simulink model "spec2in_8192ch_1080mhz"  (R2013b) (continued)
== system spec2in_8192ch_1080mhz/fft_wideband_real0/fft_direct == (continued)
  butterfly3_2 : Subsystem  AncestorBlock=casper_library_ffts/butterfly_direct  mask(butterfly_direct){n_inputs=1, biplex=off, FFTSize=14, Coeffs=[ 2 4098 2050 6146 1026 5122 3074 7170 514 4610 2562 6658 1538 5634 3586 7682 258 4354 2306 6402 1282 5378 3330 7426 770 4866 2818 6914 1794 5890 3842 7938 130 4226 2178 6274 1154 5250 3202 7298 642 4738 2690 6786 1666 5762 3714 7810 386 4482 2434 6530 1410 5506 3458 7554 898 4994 2946 7042 1922 6018 3970 8066 66 4162 2114 6210 1090 5186 3138 7234 578 4674 2626 6722 1602 5698 3650 7746 322 4418 2370 6466 1346 5442 3394 7490 834 4930 2882 6978 1858 5954 3906 8002 194 4290 2242 6338 1218 5314 3266 7362 706 4802 2754 6850 1730 5826 3778 7874 450 4546 2498 6594 1474 5570 3522 7618 962 5058 3010 7106 1986 6082 4034 8130 34 4130 2082 6178 1058 5154 3106 7202 546 4642 2594 6690 1570 5666 3618 7714 290 4386 2338 6434 1314 5410 3362 7458 802 4898 2850 6946 1826 5922 3874 7970 162 4258 2210 6306 1186 5282 3234 7330 674 4770 2722 6818 1698 5794 3746 7842 418 4514 2466 6562 1442 5538 3490 7586 930 5026 2978 7074 1954 6050 4002 8098 98 4194 2146 6242 1122 5218 3170 7266 610 4706 2658 6754 1634 5730 3682 7778 354 4450 2402 6498 1378 5474 3426 7522 866 4962 2914 7010 1890 5986 3938 8034 226 4322 2274 6370 1250 5346 3298 7394 738 4834 2786 6882 1762 5858 3810 7906 482 4578 2530 6626 1506 5602 3554 7650 994 5090 3042 7138 2018 6114 4066 8162 18 4114 2066 6162 1042 5138 3090 7186 530 4626 2578 6674 1554 5650 3602 7698 274 4370 2322 6418 1298 5394 3346 7442 786 4882 2834 6930 1810 5906 3858 7954 146 4242 2194 6290 1170 5266 3218 7314 658 4754 2706 6802 1682 5778 3730 7826 402 4498 2450 6546 1426 5522 3474 7570 914 5010 2962 7058 1938 6034 3986 8082 82 4178 2130 6226 1106 5202 3154 7250 594 4690 2642 6738 1618 5714 3666 7762 338 4434 2386 6482 1362 5458 3410 7506 850 4946 2898 6994 1874 5970 3922 8018 210 4306 2258 6354 1234 5330 3282 7378 722 4818 2770 6866 1746 5842 3794 7890 466 4562 2514 6610 1490 5586 3538 7634 978 5074 3026 7122 2002 6098 4050 8146 50 4146 2098 6194 1074 5170 3122 7218 562 4658 2610 6706 1586 5682 3634 7730 306 4402 2354 6450 1330 5426 3378 7474 818 4914 2866 6962 1842 5938 3890 7986 178 4274 2226 6322 1202 5298 3250 7346 690 4786 2738 6834 1714 5810 3762 7858 434 4530 2482 6578 1458 5554 3506 7602 946 5042 2994 7090 1970 6066 4018 8114 114 4210 2162 6258 1138 5234 3186 7282 626 4722 2674 6770 1650 5746 3698 7794 370 4466 2418 6514 1394 5490 3442 7538 882 4978 2930 7026 1906 6002 3954 8050 242 4338 2290 6386 1266 5362 3314 7410 754 4850 2802 6898 1778 5874 3826 7922 498 4594 2546 6642 1522 5618 3570 7666 1010 5106 3058 7154 2034 6130 4082 8178 10 4106 2058 6154 1034 5130 3082 7178 522 4618 2570 6666 1546 5642 3594 7690 266 4362 2314 6410 1290 5386 3338 7434 778 4874 2826 6922 1802 5898 3850 7946 138 4234 2186 6282 1162 5258 3210 7306 650 4746 2698 6794 1674 5770 3722 7818 394 4490 2442 6538 1418 5514 3466 7562 906 5002 2954 7050 1930 6026 3978 8074 74 4170 2122 6218 1098 5194 3146 7242 586 4682 2634 6730 1610 5706 3658 7754 330 4426 2378 6474 1354 5450 3402 7498 842 4938 2890 6986 1866 5962 3914 8010 202 4298 2250 6346 1226 5322 3274 7370 714 4810 2762 6858 1738 5834 3786 7882 458 4554 2506 6602 1482 5578 3530 7626 970 5066 3018 7114 1994 6090 4042 8138 42 4138 2090 6186 1066 5162 3114 7210 554 4650 2602 6698 1578 5674 3626 7722 298 4394 2346 6442 1322 5418 3370 7466 810 4906 2858 6954 1834 5930 3882 7978 170 4266 2218 6314 1194 5290 3242 7338 682 4778 2730 6826 1706 5802 3754 7850 426 4522 2474 6570 1450 5546 3498 7594 938 5034 2986 7082 1962 6058 4010 8106 106 4202 2154 6250 1130 5226 3178 7274 618 4714 2666 6762 1642 5738 3690 7786 362 4458 2410 6506 1386 5482 3434 7530 874 4970 2922 7018 1898 5994 3946 8042 234 4330 2282 6378 1258 5354 3306 7402 746 4842 2794 6890 1770 5866 3818 7914 490 4586 2538 6634 1514 5610 3562 7658 1002 5098 3050 7146 2026 6122 4074 8170 26 4122 2074 6170 1050 5146 3098 7194 538 4634 2586 6682 1562 5658 3610 7706 282 4378 2330 6426 1306 5402 3354 7450 794 4890 2842 6938 1818 5914 3866 7962 154 4250 2202 6298 1178 5274 3226 7322 666 4762 2714 6810 1690 5786 3738 7834 410 4506 2458 6554 1434 5530 ...(+984 chars)], StepPeriod=0, coeff_bit_width=18, input_bit_width=18, bin_pt_in=17, downshift=off, async=off, add_latency=1, mult_latency=2, bram_latency=2, conv_latency=1, quantization=Round  (unbiased: +/- Inf), overflow=Wrap, coeffs_bit_limit=9, coeff_sharing=off, coeff_decimation=off, coeff_generation=off, cal_bits=1, n_bits_rotation=25, max_fanout=4, use_hdl=on, use_embedded=off, bitgrowth=off, hardcode_shifts=off, dsp48_adders=off}
      mask prompts: n_inputs "number inputs"; biplex "Used in biplex FFT"; FFTSize "Size of FFT: (2^? pts)"; Coeffs "Coefficients: (0 to 2^(FFTSize-1)-1)"; StepPeriod "Coefficient Step Period: (2^?)"; coeff_bit_width "Coefficient Bit Width"; input_bit_width "Input Bit Width"; bin_pt_in "Input binary point"; async "asynchronous operation"; add_latency "Add Latency"; mult_latency "Mult Latency"; bram_latency "BRAM Latency"; conv_latency "Convert latency"; quantization "Quantization Behavior"; overflow "Overflow Behavior"; coeffs_bit_limit "Number bits above which to store stage's coefficients in BRAM (2^? bits)"; coeff_sharing "BRAM sharing in coeff storage"; coeff_decimation "Store a fraction of coeff factors where useful"; coeff_generation "Generate coeffs with multipliers where useful"; cal_bits "Number calibration locations when generating coeffs (2^?)"; n_bits_rotation "Feedback rotation vector resolution"; max_fanout "Maximum fanout"; use_hdl "Use behavioural HDL for multipliers"; use_embedded "Use embedded multipliers"; bitgrowth "Bit growth instead of shifting"; hardcode_shifts "Hardcode shift schedule"; dsp48_adders "Use DSP48s for adders"
      mask help: FFT internal block.
      mask init: same as spec2in_8192ch_1080mhz/fft_wideband_real0/fft_direct/butterfly0_0
      settings: AttributesFormatString=twiddle_general
      mask TypeOptions quantization: Truncate | Round  (unbiased: +/- Inf) | Round  (unbiased: Even Values)
      mask TypeOptions overflow: Wrap | Saturate | Error
      mask Callback use_hdl:
      | % make mutually exclusive with use_embedded.
      | 
      | use_hdl = get_param(gcb, 'use_hdl');
      | if strcmp(use_hdl, 'on'),
      |     set_param_state(gcb, 'use_embedded', 'off');
      | else
      |     set_param_state(gcb, 'use_embedded', 'on');
      | end
      mask Callback use_embedded:
      | % make mutually exclusive with use_hdl.
      | 
      | use_hdl = get_param(gcb, 'use_embedded');
      | if strcmp(use_hdl, 'on'),
      |     set_param_state(gcb, 'use_hdl', 'off');
      | else
      |     set_param_state(gcb, 'use_hdl', 'on');
      | end
      mask Callback hardcode_shifts:
      | hardcode_shifts = get_param(gcb, 'hardcode_shifts');
      | set_param_state(gcb, 'downshift', hardcode_shifts);
      mask Callback dsp48_adders: %fft_callback_dsp48_adders(gcb);
  butterfly3_3 : Subsystem  AncestorBlock=casper_library_ffts/butterfly_direct  mask(butterfly_direct){n_inputs=1, biplex=off, FFTSize=14, Coeffs=[ 3 4099 2051 6147 1027 5123 3075 7171 515 4611 2563 6659 1539 5635 3587 7683 259 4355 2307 6403 1283 5379 3331 7427 771 4867 2819 6915 1795 5891 3843 7939 131 4227 2179 6275 1155 5251 3203 7299 643 4739 2691 6787 1667 5763 3715 7811 387 4483 2435 6531 1411 5507 3459 7555 899 4995 2947 7043 1923 6019 3971 8067 67 4163 2115 6211 1091 5187 3139 7235 579 4675 2627 6723 1603 5699 3651 7747 323 4419 2371 6467 1347 5443 3395 7491 835 4931 2883 6979 1859 5955 3907 8003 195 4291 2243 6339 1219 5315 3267 7363 707 4803 2755 6851 1731 5827 3779 7875 451 4547 2499 6595 1475 5571 3523 7619 963 5059 3011 7107 1987 6083 4035 8131 35 4131 2083 6179 1059 5155 3107 7203 547 4643 2595 6691 1571 5667 3619 7715 291 4387 2339 6435 1315 5411 3363 7459 803 4899 2851 6947 1827 5923 3875 7971 163 4259 2211 6307 1187 5283 3235 7331 675 4771 2723 6819 1699 5795 3747 7843 419 4515 2467 6563 1443 5539 3491 7587 931 5027 2979 7075 1955 6051 4003 8099 99 4195 2147 6243 1123 5219 3171 7267 611 4707 2659 6755 1635 5731 3683 7779 355 4451 2403 6499 1379 5475 3427 7523 867 4963 2915 7011 1891 5987 3939 8035 227 4323 2275 6371 1251 5347 3299 7395 739 4835 2787 6883 1763 5859 3811 7907 483 4579 2531 6627 1507 5603 3555 7651 995 5091 3043 7139 2019 6115 4067 8163 19 4115 2067 6163 1043 5139 3091 7187 531 4627 2579 6675 1555 5651 3603 7699 275 4371 2323 6419 1299 5395 3347 7443 787 4883 2835 6931 1811 5907 3859 7955 147 4243 2195 6291 1171 5267 3219 7315 659 4755 2707 6803 1683 5779 3731 7827 403 4499 2451 6547 1427 5523 3475 7571 915 5011 2963 7059 1939 6035 3987 8083 83 4179 2131 6227 1107 5203 3155 7251 595 4691 2643 6739 1619 5715 3667 7763 339 4435 2387 6483 1363 5459 3411 7507 851 4947 2899 6995 1875 5971 3923 8019 211 4307 2259 6355 1235 5331 3283 7379 723 4819 2771 6867 1747 5843 3795 7891 467 4563 2515 6611 1491 5587 3539 7635 979 5075 3027 7123 2003 6099 4051 8147 51 4147 2099 6195 1075 5171 3123 7219 563 4659 2611 6707 1587 5683 3635 7731 307 4403 2355 6451 1331 5427 3379 7475 819 4915 2867 6963 1843 5939 3891 7987 179 4275 2227 6323 1203 5299 3251 7347 691 4787 2739 6835 1715 5811 3763 7859 435 4531 2483 6579 1459 5555 3507 7603 947 5043 2995 7091 1971 6067 4019 8115 115 4211 2163 6259 1139 5235 3187 7283 627 4723 2675 6771 1651 5747 3699 7795 371 4467 2419 6515 1395 5491 3443 7539 883 4979 2931 7027 1907 6003 3955 8051 243 4339 2291 6387 1267 5363 3315 7411 755 4851 2803 6899 1779 5875 3827 7923 499 4595 2547 6643 1523 5619 3571 7667 1011 5107 3059 7155 2035 6131 4083 8179 11 4107 2059 6155 1035 5131 3083 7179 523 4619 2571 6667 1547 5643 3595 7691 267 4363 2315 6411 1291 5387 3339 7435 779 4875 2827 6923 1803 5899 3851 7947 139 4235 2187 6283 1163 5259 3211 7307 651 4747 2699 6795 1675 5771 3723 7819 395 4491 2443 6539 1419 5515 3467 7563 907 5003 2955 7051 1931 6027 3979 8075 75 4171 2123 6219 1099 5195 3147 7243 587 4683 2635 6731 1611 5707 3659 7755 331 4427 2379 6475 1355 5451 3403 7499 843 4939 2891 6987 1867 5963 3915 8011 203 4299 2251 6347 1227 5323 3275 7371 715 4811 2763 6859 1739 5835 3787 7883 459 4555 2507 6603 1483 5579 3531 7627 971 5067 3019 7115 1995 6091 4043 8139 43 4139 2091 6187 1067 5163 3115 7211 555 4651 2603 6699 1579 5675 3627 7723 299 4395 2347 6443 1323 5419 3371 7467 811 4907 2859 6955 1835 5931 3883 7979 171 4267 2219 6315 1195 5291 3243 7339 683 4779 2731 6827 1707 5803 3755 7851 427 4523 2475 6571 1451 5547 3499 7595 939 5035 2987 7083 1963 6059 4011 8107 107 4203 2155 6251 1131 5227 3179 7275 619 4715 2667 6763 1643 5739 3691 7787 363 4459 2411 6507 1387 5483 3435 7531 875 4971 2923 7019 1899 5995 3947 8043 235 4331 2283 6379 1259 5355 3307 7403 747 4843 2795 6891 1771 5867 3819 7915 491 4587 2539 6635 1515 5611 3563 7659 1003 5099 3051 7147 2027 6123 4075 8171 27 4123 2075 6171 1051 5147 3099 7195 539 4635 2587 6683 1563 5659 3611 7707 283 4379 2331 6427 1307 5403 3355 7451 795 4891 2843 6939 1819 5915 3867 7963 155 4251 2203 6299 1179 5275 3227 7323 667 4763 2715 6811 1691 5787 3739 7835 411 4507 2459 6555 1435 5531 ...(+984 chars)], StepPeriod=0, coeff_bit_width=18, input_bit_width=18, bin_pt_in=17, downshift=off, async=off, add_latency=1, mult_latency=2, bram_latency=2, conv_latency=1, quantization=Round  (unbiased: +/- Inf), overflow=Wrap, coeffs_bit_limit=9, coeff_sharing=off, coeff_decimation=off, coeff_generation=off, cal_bits=1, n_bits_rotation=25, max_fanout=4, use_hdl=on, use_embedded=off, bitgrowth=off, hardcode_shifts=off, dsp48_adders=off}
      mask prompts: n_inputs "number inputs"; biplex "Used in biplex FFT"; FFTSize "Size of FFT: (2^? pts)"; Coeffs "Coefficients: (0 to 2^(FFTSize-1)-1)"; StepPeriod "Coefficient Step Period: (2^?)"; coeff_bit_width "Coefficient Bit Width"; input_bit_width "Input Bit Width"; bin_pt_in "Input binary point"; async "asynchronous operation"; add_latency "Add Latency"; mult_latency "Mult Latency"; bram_latency "BRAM Latency"; conv_latency "Convert latency"; quantization "Quantization Behavior"; overflow "Overflow Behavior"; coeffs_bit_limit "Number bits above which to store stage's coefficients in BRAM (2^? bits)"; coeff_sharing "BRAM sharing in coeff storage"; coeff_decimation "Store a fraction of coeff factors where useful"; coeff_generation "Generate coeffs with multipliers where useful"; cal_bits "Number calibration locations when generating coeffs (2^?)"; n_bits_rotation "Feedback rotation vector resolution"; max_fanout "Maximum fanout"; use_hdl "Use behavioural HDL for multipliers"; use_embedded "Use embedded multipliers"; bitgrowth "Bit growth instead of shifting"; hardcode_shifts "Hardcode shift schedule"; dsp48_adders "Use DSP48s for adders"
      mask help: FFT internal block.
      mask init: same as spec2in_8192ch_1080mhz/fft_wideband_real0/fft_direct/butterfly0_0
      settings: AttributesFormatString=twiddle_general
      mask TypeOptions quantization: Truncate | Round  (unbiased: +/- Inf) | Round  (unbiased: Even Values)
      mask TypeOptions overflow: Wrap | Saturate | Error
      mask Callback use_hdl:
      | % make mutually exclusive with use_embedded.
      | 
      | use_hdl = get_param(gcb, 'use_hdl');
      | if strcmp(use_hdl, 'on'),
      |     set_param_state(gcb, 'use_embedded', 'off');
      | else
      |     set_param_state(gcb, 'use_embedded', 'on');
      | end
      mask Callback use_embedded:
      | % make mutually exclusive with use_hdl.
      | 
      | use_hdl = get_param(gcb, 'use_embedded');
      | if strcmp(use_hdl, 'on'),
      |     set_param_state(gcb, 'use_hdl', 'off');
      | else
      |     set_param_state(gcb, 'use_hdl', 'on');
      | end
      mask Callback hardcode_shifts:
      | hardcode_shifts = get_param(gcb, 'hardcode_shifts');
      | set_param_state(gcb, 'downshift', hardcode_shifts);
      mask Callback dsp48_adders: %fft_callback_dsp48_adders(gcb);
  butterfly3_4 : Subsystem  AncestorBlock=casper_library_ffts/butterfly_direct  mask(butterfly_direct){n_inputs=1, biplex=off, FFTSize=14, Coeffs=[ 4 4100 2052 6148 1028 5124 3076 7172 516 4612 2564 6660 1540 5636 3588 7684 260 4356 2308 6404 1284 5380 3332 7428 772 4868 2820 6916 1796 5892 3844 7940 132 4228 2180 6276 1156 5252 3204 7300 644 4740 2692 6788 1668 5764 3716 7812 388 4484 2436 6532 1412 5508 3460 7556 900 4996 2948 7044 1924 6020 3972 8068 68 4164 2116 6212 1092 5188 3140 7236 580 4676 2628 6724 1604 5700 3652 7748 324 4420 2372 6468 1348 5444 3396 7492 836 4932 2884 6980 1860 5956 3908 8004 196 4292 2244 6340 1220 5316 3268 7364 708 4804 2756 6852 1732 5828 3780 7876 452 4548 2500 6596 1476 5572 3524 7620 964 5060 3012 7108 1988 6084 4036 8132 36 4132 2084 6180 1060 5156 3108 7204 548 4644 2596 6692 1572 5668 3620 7716 292 4388 2340 6436 1316 5412 3364 7460 804 4900 2852 6948 1828 5924 3876 7972 164 4260 2212 6308 1188 5284 3236 7332 676 4772 2724 6820 1700 5796 3748 7844 420 4516 2468 6564 1444 5540 3492 7588 932 5028 2980 7076 1956 6052 4004 8100 100 4196 2148 6244 1124 5220 3172 7268 612 4708 2660 6756 1636 5732 3684 7780 356 4452 2404 6500 1380 5476 3428 7524 868 4964 2916 7012 1892 5988 3940 8036 228 4324 2276 6372 1252 5348 3300 7396 740 4836 2788 6884 1764 5860 3812 7908 484 4580 2532 6628 1508 5604 3556 7652 996 5092 3044 7140 2020 6116 4068 8164 20 4116 2068 6164 1044 5140 3092 7188 532 4628 2580 6676 1556 5652 3604 7700 276 4372 2324 6420 1300 5396 3348 7444 788 4884 2836 6932 1812 5908 3860 7956 148 4244 2196 6292 1172 5268 3220 7316 660 4756 2708 6804 1684 5780 3732 7828 404 4500 2452 6548 1428 5524 3476 7572 916 5012 2964 7060 1940 6036 3988 8084 84 4180 2132 6228 1108 5204 3156 7252 596 4692 2644 6740 1620 5716 3668 7764 340 4436 2388 6484 1364 5460 3412 7508 852 4948 2900 6996 1876 5972 3924 8020 212 4308 2260 6356 1236 5332 3284 7380 724 4820 2772 6868 1748 5844 3796 7892 468 4564 2516 6612 1492 5588 3540 7636 980 5076 3028 7124 2004 6100 4052 8148 52 4148 2100 6196 1076 5172 3124 7220 564 4660 2612 6708 1588 5684 3636 7732 308 4404 2356 6452 1332 5428 3380 7476 820 4916 2868 6964 1844 5940 3892 7988 180 4276 2228 6324 1204 5300 3252 7348 692 4788 2740 6836 1716 5812 3764 7860 436 4532 2484 6580 1460 5556 3508 7604 948 5044 2996 7092 1972 6068 4020 8116 116 4212 2164 6260 1140 5236 3188 7284 628 4724 2676 6772 1652 5748 3700 7796 372 4468 2420 6516 1396 5492 3444 7540 884 4980 2932 7028 1908 6004 3956 8052 244 4340 2292 6388 1268 5364 3316 7412 756 4852 2804 6900 1780 5876 3828 7924 500 4596 2548 6644 1524 5620 3572 7668 1012 5108 3060 7156 2036 6132 4084 8180 12 4108 2060 6156 1036 5132 3084 7180 524 4620 2572 6668 1548 5644 3596 7692 268 4364 2316 6412 1292 5388 3340 7436 780 4876 2828 6924 1804 5900 3852 7948 140 4236 2188 6284 1164 5260 3212 7308 652 4748 2700 6796 1676 5772 3724 7820 396 4492 2444 6540 1420 5516 3468 7564 908 5004 2956 7052 1932 6028 3980 8076 76 4172 2124 6220 1100 5196 3148 7244 588 4684 2636 6732 1612 5708 3660 7756 332 4428 2380 6476 1356 5452 3404 7500 844 4940 2892 6988 1868 5964 3916 8012 204 4300 2252 6348 1228 5324 3276 7372 716 4812 2764 6860 1740 5836 3788 7884 460 4556 2508 6604 1484 5580 3532 7628 972 5068 3020 7116 1996 6092 4044 8140 44 4140 2092 6188 1068 5164 3116 7212 556 4652 2604 6700 1580 5676 3628 7724 300 4396 2348 6444 1324 5420 3372 7468 812 4908 2860 6956 1836 5932 3884 7980 172 4268 2220 6316 1196 5292 3244 7340 684 4780 2732 6828 1708 5804 3756 7852 428 4524 2476 6572 1452 5548 3500 7596 940 5036 2988 7084 1964 6060 4012 8108 108 4204 2156 6252 1132 5228 3180 7276 620 4716 2668 6764 1644 5740 3692 7788 364 4460 2412 6508 1388 5484 3436 7532 876 4972 2924 7020 1900 5996 3948 8044 236 4332 2284 6380 1260 5356 3308 7404 748 4844 2796 6892 1772 5868 3820 7916 492 4588 2540 6636 1516 5612 3564 7660 1004 5100 3052 7148 2028 6124 4076 8172 28 4124 2076 6172 1052 5148 3100 7196 540 4636 2588 6684 1564 5660 3612 7708 284 4380 2332 6428 1308 5404 3356 7452 796 4892 2844 6940 1820 5916 3868 7964 156 4252 2204 6300 1180 5276 3228 7324 668 4764 2716 6812 1692 5788 3740 7836 412 4508 2460 6556 1436 5532 ...(+984 chars)], StepPeriod=0, coeff_bit_width=18, input_bit_width=18, bin_pt_in=17, downshift=off, async=off, add_latency=1, mult_latency=2, bram_latency=2, conv_latency=1, quantization=Round  (unbiased: +/- Inf), overflow=Wrap, coeffs_bit_limit=9, coeff_sharing=off, coeff_decimation=off, coeff_generation=off, cal_bits=1, n_bits_rotation=25, max_fanout=4, use_hdl=on, use_embedded=off, bitgrowth=off, hardcode_shifts=off, dsp48_adders=off}
      mask prompts: n_inputs "number inputs"; biplex "Used in biplex FFT"; FFTSize "Size of FFT: (2^? pts)"; Coeffs "Coefficients: (0 to 2^(FFTSize-1)-1)"; StepPeriod "Coefficient Step Period: (2^?)"; coeff_bit_width "Coefficient Bit Width"; input_bit_width "Input Bit Width"; bin_pt_in "Input binary point"; async "asynchronous operation"; add_latency "Add Latency"; mult_latency "Mult Latency"; bram_latency "BRAM Latency"; conv_latency "Convert latency"; quantization "Quantization Behavior"; overflow "Overflow Behavior"; coeffs_bit_limit "Number bits above which to store stage's coefficients in BRAM (2^? bits)"; coeff_sharing "BRAM sharing in coeff storage"; coeff_decimation "Store a fraction of coeff factors where useful"; coeff_generation "Generate coeffs with multipliers where useful"; cal_bits "Number calibration locations when generating coeffs (2^?)"; n_bits_rotation "Feedback rotation vector resolution"; max_fanout "Maximum fanout"; use_hdl "Use behavioural HDL for multipliers"; use_embedded "Use embedded multipliers"; bitgrowth "Bit growth instead of shifting"; hardcode_shifts "Hardcode shift schedule"; dsp48_adders "Use DSP48s for adders"
      mask help: FFT internal block.
      mask init: same as spec2in_8192ch_1080mhz/fft_wideband_real0/fft_direct/butterfly0_0
      settings: AttributesFormatString=twiddle_general
      mask TypeOptions quantization: Truncate | Round  (unbiased: +/- Inf) | Round  (unbiased: Even Values)
      mask TypeOptions overflow: Wrap | Saturate | Error
      mask Callback use_hdl:
      | % make mutually exclusive with use_embedded.
      | 
      | use_hdl = get_param(gcb, 'use_hdl');
      | if strcmp(use_hdl, 'on'),
      |     set_param_state(gcb, 'use_embedded', 'off');
      | else
      |     set_param_state(gcb, 'use_embedded', 'on');
      | end
      mask Callback use_embedded:
      | % make mutually exclusive with use_hdl.
      | 
      | use_hdl = get_param(gcb, 'use_embedded');
      | if strcmp(use_hdl, 'on'),
      |     set_param_state(gcb, 'use_hdl', 'off');
      | else
      |     set_param_state(gcb, 'use_hdl', 'on');
      | end
      mask Callback hardcode_shifts:
      | hardcode_shifts = get_param(gcb, 'hardcode_shifts');
      | set_param_state(gcb, 'downshift', hardcode_shifts);
      mask Callback dsp48_adders: %fft_callback_dsp48_adders(gcb);
  butterfly3_5 : Subsystem  AncestorBlock=casper_library_ffts/butterfly_direct  mask(butterfly_direct){n_inputs=1, biplex=off, FFTSize=14, Coeffs=[ 5 4101 2053 6149 1029 5125 3077 7173 517 4613 2565 6661 1541 5637 3589 7685 261 4357 2309 6405 1285 5381 3333 7429 773 4869 2821 6917 1797 5893 3845 7941 133 4229 2181 6277 1157 5253 3205 7301 645 4741 2693 6789 1669 5765 3717 7813 389 4485 2437 6533 1413 5509 3461 7557 901 4997 2949 7045 1925 6021 3973 8069 69 4165 2117 6213 1093 5189 3141 7237 581 4677 2629 6725 1605 5701 3653 7749 325 4421 2373 6469 1349 5445 3397 7493 837 4933 2885 6981 1861 5957 3909 8005 197 4293 2245 6341 1221 5317 3269 7365 709 4805 2757 6853 1733 5829 3781 7877 453 4549 2501 6597 1477 5573 3525 7621 965 5061 3013 7109 1989 6085 4037 8133 37 4133 2085 6181 1061 5157 3109 7205 549 4645 2597 6693 1573 5669 3621 7717 293 4389 2341 6437 1317 5413 3365 7461 805 4901 2853 6949 1829 5925 3877 7973 165 4261 2213 6309 1189 5285 3237 7333 677 4773 2725 6821 1701 5797 3749 7845 421 4517 2469 6565 1445 5541 3493 7589 933 5029 2981 7077 1957 6053 4005 8101 101 4197 2149 6245 1125 5221 3173 7269 613 4709 2661 6757 1637 5733 3685 7781 357 4453 2405 6501 1381 5477 3429 7525 869 4965 2917 7013 1893 5989 3941 8037 229 4325 2277 6373 1253 5349 3301 7397 741 4837 2789 6885 1765 5861 3813 7909 485 4581 2533 6629 1509 5605 3557 7653 997 5093 3045 7141 2021 6117 4069 8165 21 4117 2069 6165 1045 5141 3093 7189 533 4629 2581 6677 1557 5653 3605 7701 277 4373 2325 6421 1301 5397 3349 7445 789 4885 2837 6933 1813 5909 3861 7957 149 4245 2197 6293 1173 5269 3221 7317 661 4757 2709 6805 1685 5781 3733 7829 405 4501 2453 6549 1429 5525 3477 7573 917 5013 2965 7061 1941 6037 3989 8085 85 4181 2133 6229 1109 5205 3157 7253 597 4693 2645 6741 1621 5717 3669 7765 341 4437 2389 6485 1365 5461 3413 7509 853 4949 2901 6997 1877 5973 3925 8021 213 4309 2261 6357 1237 5333 3285 7381 725 4821 2773 6869 1749 5845 3797 7893 469 4565 2517 6613 1493 5589 3541 7637 981 5077 3029 7125 2005 6101 4053 8149 53 4149 2101 6197 1077 5173 3125 7221 565 4661 2613 6709 1589 5685 3637 7733 309 4405 2357 6453 1333 5429 3381 7477 821 4917 2869 6965 1845 5941 3893 7989 181 4277 2229 6325 1205 5301 3253 7349 693 4789 2741 6837 1717 5813 3765 7861 437 4533 2485 6581 1461 5557 3509 7605 949 5045 2997 7093 1973 6069 4021 8117 117 4213 2165 6261 1141 5237 3189 7285 629 4725 2677 6773 1653 5749 3701 7797 373 4469 2421 6517 1397 5493 3445 7541 885 4981 2933 7029 1909 6005 3957 8053 245 4341 2293 6389 1269 5365 3317 7413 757 4853 2805 6901 1781 5877 3829 7925 501 4597 2549 6645 1525 5621 3573 7669 1013 5109 3061 7157 2037 6133 4085 8181 13 4109 2061 6157 1037 5133 3085 7181 525 4621 2573 6669 1549 5645 3597 7693 269 4365 2317 6413 1293 5389 3341 7437 781 4877 2829 6925 1805 5901 3853 7949 141 4237 2189 6285 1165 5261 3213 7309 653 4749 2701 6797 1677 5773 3725 7821 397 4493 2445 6541 1421 5517 3469 7565 909 5005 2957 7053 1933 6029 3981 8077 77 4173 2125 6221 1101 5197 3149 7245 589 4685 2637 6733 1613 5709 3661 7757 333 4429 2381 6477 1357 5453 3405 7501 845 4941 2893 6989 1869 5965 3917 8013 205 4301 2253 6349 1229 5325 3277 7373 717 4813 2765 6861 1741 5837 3789 7885 461 4557 2509 6605 1485 5581 3533 7629 973 5069 3021 7117 1997 6093 4045 8141 45 4141 2093 6189 1069 5165 3117 7213 557 4653 2605 6701 1581 5677 3629 7725 301 4397 2349 6445 1325 5421 3373 7469 813 4909 2861 6957 1837 5933 3885 7981 173 4269 2221 6317 1197 5293 3245 7341 685 4781 2733 6829 1709 5805 3757 7853 429 4525 2477 6573 1453 5549 3501 7597 941 5037 2989 7085 1965 6061 4013 8109 109 4205 2157 6253 1133 5229 3181 7277 621 4717 2669 6765 1645 5741 3693 7789 365 4461 2413 6509 1389 5485 3437 7533 877 4973 2925 7021 1901 5997 3949 8045 237 4333 2285 6381 1261 5357 3309 7405 749 4845 2797 6893 1773 5869 3821 7917 493 4589 2541 6637 1517 5613 3565 7661 1005 5101 3053 7149 2029 6125 4077 8173 29 4125 2077 6173 1053 5149 3101 7197 541 4637 2589 6685 1565 5661 3613 7709 285 4381 2333 6429 1309 5405 3357 7453 797 4893 2845 6941 1821 5917 3869 7965 157 4253 2205 6301 1181 5277 3229 7325 669 4765 2717 6813 1693 5789 3741 7837 413 4509 2461 6557 1437 5533 ...(+984 chars)], StepPeriod=0, coeff_bit_width=18, input_bit_width=18, bin_pt_in=17, downshift=off, async=off, add_latency=1, mult_latency=2, bram_latency=2, conv_latency=1, quantization=Round  (unbiased: +/- Inf), overflow=Wrap, coeffs_bit_limit=9, coeff_sharing=off, coeff_decimation=off, coeff_generation=off, cal_bits=1, n_bits_rotation=25, max_fanout=4, use_hdl=on, use_embedded=off, bitgrowth=off, hardcode_shifts=off, dsp48_adders=off}
      mask prompts: n_inputs "number inputs"; biplex "Used in biplex FFT"; FFTSize "Size of FFT: (2^? pts)"; Coeffs "Coefficients: (0 to 2^(FFTSize-1)-1)"; StepPeriod "Coefficient Step Period: (2^?)"; coeff_bit_width "Coefficient Bit Width"; input_bit_width "Input Bit Width"; bin_pt_in "Input binary point"; async "asynchronous operation"; add_latency "Add Latency"; mult_latency "Mult Latency"; bram_latency "BRAM Latency"; conv_latency "Convert latency"; quantization "Quantization Behavior"; overflow "Overflow Behavior"; coeffs_bit_limit "Number bits above which to store stage's coefficients in BRAM (2^? bits)"; coeff_sharing "BRAM sharing in coeff storage"; coeff_decimation "Store a fraction of coeff factors where useful"; coeff_generation "Generate coeffs with multipliers where useful"; cal_bits "Number calibration locations when generating coeffs (2^?)"; n_bits_rotation "Feedback rotation vector resolution"; max_fanout "Maximum fanout"; use_hdl "Use behavioural HDL for multipliers"; use_embedded "Use embedded multipliers"; bitgrowth "Bit growth instead of shifting"; hardcode_shifts "Hardcode shift schedule"; dsp48_adders "Use DSP48s for adders"
      mask help: FFT internal block.
      mask init: same as spec2in_8192ch_1080mhz/fft_wideband_real0/fft_direct/butterfly0_0
      settings: AttributesFormatString=twiddle_general
      mask TypeOptions quantization: Truncate | Round  (unbiased: +/- Inf) | Round  (unbiased: Even Values)
      mask TypeOptions overflow: Wrap | Saturate | Error
      mask Callback use_hdl:
      | % make mutually exclusive with use_embedded.
      | 
      | use_hdl = get_param(gcb, 'use_hdl');
      | if strcmp(use_hdl, 'on'),
      |     set_param_state(gcb, 'use_embedded', 'off');
      | else
      |     set_param_state(gcb, 'use_embedded', 'on');
      | end
      mask Callback use_embedded:
      | % make mutually exclusive with use_hdl.
      | 
      | use_hdl = get_param(gcb, 'use_embedded');
      | if strcmp(use_hdl, 'on'),
      |     set_param_state(gcb, 'use_hdl', 'off');
      | else
      |     set_param_state(gcb, 'use_hdl', 'on');
      | end
      mask Callback hardcode_shifts:
      | hardcode_shifts = get_param(gcb, 'hardcode_shifts');
      | set_param_state(gcb, 'downshift', hardcode_shifts);
      mask Callback dsp48_adders: %fft_callback_dsp48_adders(gcb);
  butterfly3_6 : Subsystem  AncestorBlock=casper_library_ffts/butterfly_direct  mask(butterfly_direct){n_inputs=1, biplex=off, FFTSize=14, Coeffs=[ 6 4102 2054 6150 1030 5126 3078 7174 518 4614 2566 6662 1542 5638 3590 7686 262 4358 2310 6406 1286 5382 3334 7430 774 4870 2822 6918 1798 5894 3846 7942 134 4230 2182 6278 1158 5254 3206 7302 646 4742 2694 6790 1670 5766 3718 7814 390 4486 2438 6534 1414 5510 3462 7558 902 4998 2950 7046 1926 6022 3974 8070 70 4166 2118 6214 1094 5190 3142 7238 582 4678 2630 6726 1606 5702 3654 7750 326 4422 2374 6470 1350 5446 3398 7494 838 4934 2886 6982 1862 5958 3910 8006 198 4294 2246 6342 1222 5318 3270 7366 710 4806 2758 6854 1734 5830 3782 7878 454 4550 2502 6598 1478 5574 3526 7622 966 5062 3014 7110 1990 6086 4038 8134 38 4134 2086 6182 1062 5158 3110 7206 550 4646 2598 6694 1574 5670 3622 7718 294 4390 2342 6438 1318 5414 3366 7462 806 4902 2854 6950 1830 5926 3878 7974 166 4262 2214 6310 1190 5286 3238 7334 678 4774 2726 6822 1702 5798 3750 7846 422 4518 2470 6566 1446 5542 3494 7590 934 5030 2982 7078 1958 6054 4006 8102 102 4198 2150 6246 1126 5222 3174 7270 614 4710 2662 6758 1638 5734 3686 7782 358 4454 2406 6502 1382 5478 3430 7526 870 4966 2918 7014 1894 5990 3942 8038 230 4326 2278 6374 1254 5350 3302 7398 742 4838 2790 6886 1766 5862 3814 7910 486 4582 2534 6630 1510 5606 3558 7654 998 5094 3046 7142 2022 6118 4070 8166 22 4118 2070 6166 1046 5142 3094 7190 534 4630 2582 6678 1558 5654 3606 7702 278 4374 2326 6422 1302 5398 3350 7446 790 4886 2838 6934 1814 5910 3862 7958 150 4246 2198 6294 1174 5270 3222 7318 662 4758 2710 6806 1686 5782 3734 7830 406 4502 2454 6550 1430 5526 3478 7574 918 5014 2966 7062 1942 6038 3990 8086 86 4182 2134 6230 1110 5206 3158 7254 598 4694 2646 6742 1622 5718 3670 7766 342 4438 2390 6486 1366 5462 3414 7510 854 4950 2902 6998 1878 5974 3926 8022 214 4310 2262 6358 1238 5334 3286 7382 726 4822 2774 6870 1750 5846 3798 7894 470 4566 2518 6614 1494 5590 3542 7638 982 5078 3030 7126 2006 6102 4054 8150 54 4150 2102 6198 1078 5174 3126 7222 566 4662 2614 6710 1590 5686 3638 7734 310 4406 2358 6454 1334 5430 3382 7478 822 4918 2870 6966 1846 5942 3894 7990 182 4278 2230 6326 1206 5302 3254 7350 694 4790 2742 6838 1718 5814 3766 7862 438 4534 2486 6582 1462 5558 3510 7606 950 5046 2998 7094 1974 6070 4022 8118 118 4214 2166 6262 1142 5238 3190 7286 630 4726 2678 6774 1654 5750 3702 7798 374 4470 2422 6518 1398 5494 3446 7542 886 4982 2934 7030 1910 6006 3958 8054 246 4342 2294 6390 1270 5366 3318 7414 758 4854 2806 6902 1782 5878 3830 7926 502 4598 2550 6646 1526 5622 3574 7670 1014 5110 3062 7158 2038 6134 4086 8182 14 4110 2062 6158 1038 5134 3086 7182 526 4622 2574 6670 1550 5646 3598 7694 270 4366 2318 6414 1294 5390 3342 7438 782 4878 2830 6926 1806 5902 3854 7950 142 4238 2190 6286 1166 5262 3214 7310 654 4750 2702 6798 1678 5774 3726 7822 398 4494 2446 6542 1422 5518 3470 7566 910 5006 2958 7054 1934 6030 3982 8078 78 4174 2126 6222 1102 5198 3150 7246 590 4686 2638 6734 1614 5710 3662 7758 334 4430 2382 6478 1358 5454 3406 7502 846 4942 2894 6990 1870 5966 3918 8014 206 4302 2254 6350 1230 5326 3278 7374 718 4814 2766 6862 1742 5838 3790 7886 462 4558 2510 6606 1486 5582 3534 7630 974 5070 3022 7118 1998 6094 4046 8142 46 4142 2094 6190 1070 5166 3118 7214 558 4654 2606 6702 1582 5678 3630 7726 302 4398 2350 6446 1326 5422 3374 7470 814 4910 2862 6958 1838 5934 3886 7982 174 4270 2222 6318 1198 5294 3246 7342 686 4782 2734 6830 1710 5806 3758 7854 430 4526 2478 6574 1454 5550 3502 7598 942 5038 2990 7086 1966 6062 4014 8110 110 4206 2158 6254 1134 5230 3182 7278 622 4718 2670 6766 1646 5742 3694 7790 366 4462 2414 6510 1390 5486 3438 7534 878 4974 2926 7022 1902 5998 3950 8046 238 4334 2286 6382 1262 5358 3310 7406 750 4846 2798 6894 1774 5870 3822 7918 494 4590 2542 6638 1518 5614 3566 7662 1006 5102 3054 7150 2030 6126 4078 8174 30 4126 2078 6174 1054 5150 3102 7198 542 4638 2590 6686 1566 5662 3614 7710 286 4382 2334 6430 1310 5406 3358 7454 798 4894 2846 6942 1822 5918 3870 7966 158 4254 2206 6302 1182 5278 3230 7326 670 4766 2718 6814 1694 5790 3742 7838 414 4510 2462 6558 1438 5534 ...(+984 chars)], StepPeriod=0, coeff_bit_width=18, input_bit_width=18, bin_pt_in=17, downshift=off, async=off, add_latency=1, mult_latency=2, bram_latency=2, conv_latency=1, quantization=Round  (unbiased: +/- Inf), overflow=Wrap, coeffs_bit_limit=9, coeff_sharing=off, coeff_decimation=off, coeff_generation=off, cal_bits=1, n_bits_rotation=25, max_fanout=4, use_hdl=on, use_embedded=off, bitgrowth=off, hardcode_shifts=off, dsp48_adders=off}
      mask prompts: n_inputs "number inputs"; biplex "Used in biplex FFT"; FFTSize "Size of FFT: (2^? pts)"; Coeffs "Coefficients: (0 to 2^(FFTSize-1)-1)"; StepPeriod "Coefficient Step Period: (2^?)"; coeff_bit_width "Coefficient Bit Width"; input_bit_width "Input Bit Width"; bin_pt_in "Input binary point"; async "asynchronous operation"; add_latency "Add Latency"; mult_latency "Mult Latency"; bram_latency "BRAM Latency"; conv_latency "Convert latency"; quantization "Quantization Behavior"; overflow "Overflow Behavior"; coeffs_bit_limit "Number bits above which to store stage's coefficients in BRAM (2^? bits)"; coeff_sharing "BRAM sharing in coeff storage"; coeff_decimation "Store a fraction of coeff factors where useful"; coeff_generation "Generate coeffs with multipliers where useful"; cal_bits "Number calibration locations when generating coeffs (2^?)"; n_bits_rotation "Feedback rotation vector resolution"; max_fanout "Maximum fanout"; use_hdl "Use behavioural HDL for multipliers"; use_embedded "Use embedded multipliers"; bitgrowth "Bit growth instead of shifting"; hardcode_shifts "Hardcode shift schedule"; dsp48_adders "Use DSP48s for adders"
      mask help: FFT internal block.
      mask init: same as spec2in_8192ch_1080mhz/fft_wideband_real0/fft_direct/butterfly0_0
      settings: AttributesFormatString=twiddle_general
      mask TypeOptions quantization: Truncate | Round  (unbiased: +/- Inf) | Round  (unbiased: Even Values)
      mask TypeOptions overflow: Wrap | Saturate | Error
      mask Callback use_hdl:
      | % make mutually exclusive with use_embedded.
      | 
      | use_hdl = get_param(gcb, 'use_hdl');
      | if strcmp(use_hdl, 'on'),
      |     set_param_state(gcb, 'use_embedded', 'off');
      | else
      |     set_param_state(gcb, 'use_embedded', 'on');
      | end
      mask Callback use_embedded:
      | % make mutually exclusive with use_hdl.
      | 
      | use_hdl = get_param(gcb, 'use_embedded');
      | if strcmp(use_hdl, 'on'),
      |     set_param_state(gcb, 'use_hdl', 'off');
      | else
      |     set_param_state(gcb, 'use_hdl', 'on');
      | end
      mask Callback hardcode_shifts:
      | hardcode_shifts = get_param(gcb, 'hardcode_shifts');
      | set_param_state(gcb, 'downshift', hardcode_shifts);
      mask Callback dsp48_adders: %fft_callback_dsp48_adders(gcb);
  butterfly3_7 : Subsystem  AncestorBlock=casper_library_ffts/butterfly_direct  mask(butterfly_direct){n_inputs=1, biplex=off, FFTSize=14, Coeffs=[ 7 4103 2055 6151 1031 5127 3079 7175 519 4615 2567 6663 1543 5639 3591 7687 263 4359 2311 6407 1287 5383 3335 7431 775 4871 2823 6919 1799 5895 3847 7943 135 4231 2183 6279 1159 5255 3207 7303 647 4743 2695 6791 1671 5767 3719 7815 391 4487 2439 6535 1415 5511 3463 7559 903 4999 2951 7047 1927 6023 3975 8071 71 4167 2119 6215 1095 5191 3143 7239 583 4679 2631 6727 1607 5703 3655 7751 327 4423 2375 6471 1351 5447 3399 7495 839 4935 2887 6983 1863 5959 3911 8007 199 4295 2247 6343 1223 5319 3271 7367 711 4807 2759 6855 1735 5831 3783 7879 455 4551 2503 6599 1479 5575 3527 7623 967 5063 3015 7111 1991 6087 4039 8135 39 4135 2087 6183 1063 5159 3111 7207 551 4647 2599 6695 1575 5671 3623 7719 295 4391 2343 6439 1319 5415 3367 7463 807 4903 2855 6951 1831 5927 3879 7975 167 4263 2215 6311 1191 5287 3239 7335 679 4775 2727 6823 1703 5799 3751 7847 423 4519 2471 6567 1447 5543 3495 7591 935 5031 2983 7079 1959 6055 4007 8103 103 4199 2151 6247 1127 5223 3175 7271 615 4711 2663 6759 1639 5735 3687 7783 359 4455 2407 6503 1383 5479 3431 7527 871 4967 2919 7015 1895 5991 3943 8039 231 4327 2279 6375 1255 5351 3303 7399 743 4839 2791 6887 1767 5863 3815 7911 487 4583 2535 6631 1511 5607 3559 7655 999 5095 3047 7143 2023 6119 4071 8167 23 4119 2071 6167 1047 5143 3095 7191 535 4631 2583 6679 1559 5655 3607 7703 279 4375 2327 6423 1303 5399 3351 7447 791 4887 2839 6935 1815 5911 3863 7959 151 4247 2199 6295 1175 5271 3223 7319 663 4759 2711 6807 1687 5783 3735 7831 407 4503 2455 6551 1431 5527 3479 7575 919 5015 2967 7063 1943 6039 3991 8087 87 4183 2135 6231 1111 5207 3159 7255 599 4695 2647 6743 1623 5719 3671 7767 343 4439 2391 6487 1367 5463 3415 7511 855 4951 2903 6999 1879 5975 3927 8023 215 4311 2263 6359 1239 5335 3287 7383 727 4823 2775 6871 1751 5847 3799 7895 471 4567 2519 6615 1495 5591 3543 7639 983 5079 3031 7127 2007 6103 4055 8151 55 4151 2103 6199 1079 5175 3127 7223 567 4663 2615 6711 1591 5687 3639 7735 311 4407 2359 6455 1335 5431 3383 7479 823 4919 2871 6967 1847 5943 3895 7991 183 4279 2231 6327 1207 5303 3255 7351 695 4791 2743 6839 1719 5815 3767 7863 439 4535 2487 6583 1463 5559 3511 7607 951 5047 2999 7095 1975 6071 4023 8119 119 4215 2167 6263 1143 5239 3191 7287 631 4727 2679 6775 1655 5751 3703 7799 375 4471 2423 6519 1399 5495 3447 7543 887 4983 2935 7031 1911 6007 3959 8055 247 4343 2295 6391 1271 5367 3319 7415 759 4855 2807 6903 1783 5879 3831 7927 503 4599 2551 6647 1527 5623 3575 7671 1015 5111 3063 7159 2039 6135 4087 8183 15 4111 2063 6159 1039 5135 3087 7183 527 4623 2575 6671 1551 5647 3599 7695 271 4367 2319 6415 1295 5391 3343 7439 783 4879 2831 6927 1807 5903 3855 7951 143 4239 2191 6287 1167 5263 3215 7311 655 4751 2703 6799 1679 5775 3727 7823 399 4495 2447 6543 1423 5519 3471 7567 911 5007 2959 7055 1935 6031 3983 8079 79 4175 2127 6223 1103 5199 3151 7247 591 4687 2639 6735 1615 5711 3663 7759 335 4431 2383 6479 1359 5455 3407 7503 847 4943 2895 6991 1871 5967 3919 8015 207 4303 2255 6351 1231 5327 3279 7375 719 4815 2767 6863 1743 5839 3791 7887 463 4559 2511 6607 1487 5583 3535 7631 975 5071 3023 7119 1999 6095 4047 8143 47 4143 2095 6191 1071 5167 3119 7215 559 4655 2607 6703 1583 5679 3631 7727 303 4399 2351 6447 1327 5423 3375 7471 815 4911 2863 6959 1839 5935 3887 7983 175 4271 2223 6319 1199 5295 3247 7343 687 4783 2735 6831 1711 5807 3759 7855 431 4527 2479 6575 1455 5551 3503 7599 943 5039 2991 7087 1967 6063 4015 8111 111 4207 2159 6255 1135 5231 3183 7279 623 4719 2671 6767 1647 5743 3695 7791 367 4463 2415 6511 1391 5487 3439 7535 879 4975 2927 7023 1903 5999 3951 8047 239 4335 2287 6383 1263 5359 3311 7407 751 4847 2799 6895 1775 5871 3823 7919 495 4591 2543 6639 1519 5615 3567 7663 1007 5103 3055 7151 2031 6127 4079 8175 31 4127 2079 6175 1055 5151 3103 7199 543 4639 2591 6687 1567 5663 3615 7711 287 4383 2335 6431 1311 5407 3359 7455 799 4895 2847 6943 1823 5919 3871 7967 159 4255 2207 6303 1183 5279 3231 7327 671 4767 2719 6815 1695 5791 3743 7839 415 4511 2463 6559 1439 5535 ...(+984 chars)], StepPeriod=0, coeff_bit_width=18, input_bit_width=18, bin_pt_in=17, downshift=off, async=off, add_latency=1, mult_latency=2, bram_latency=2, conv_latency=1, quantization=Round  (unbiased: +/- Inf), overflow=Wrap, coeffs_bit_limit=9, coeff_sharing=off, coeff_decimation=off, coeff_generation=off, cal_bits=1, n_bits_rotation=25, max_fanout=4, use_hdl=on, use_embedded=off, bitgrowth=off, hardcode_shifts=off, dsp48_adders=off}
      mask prompts: n_inputs "number inputs"; biplex "Used in biplex FFT"; FFTSize "Size of FFT: (2^? pts)"; Coeffs "Coefficients: (0 to 2^(FFTSize-1)-1)"; StepPeriod "Coefficient Step Period: (2^?)"; coeff_bit_width "Coefficient Bit Width"; input_bit_width "Input Bit Width"; bin_pt_in "Input binary point"; async "asynchronous operation"; add_latency "Add Latency"; mult_latency "Mult Latency"; bram_latency "BRAM Latency"; conv_latency "Convert latency"; quantization "Quantization Behavior"; overflow "Overflow Behavior"; coeffs_bit_limit "Number bits above which to store stage's coefficients in BRAM (2^? bits)"; coeff_sharing "BRAM sharing in coeff storage"; coeff_decimation "Store a fraction of coeff factors where useful"; coeff_generation "Generate coeffs with multipliers where useful"; cal_bits "Number calibration locations when generating coeffs (2^?)"; n_bits_rotation "Feedback rotation vector resolution"; max_fanout "Maximum fanout"; use_hdl "Use behavioural HDL for multipliers"; use_embedded "Use embedded multipliers"; bitgrowth "Bit growth instead of shifting"; hardcode_shifts "Hardcode shift schedule"; dsp48_adders "Use DSP48s for adders"
      mask help: FFT internal block.
      mask init: same as spec2in_8192ch_1080mhz/fft_wideband_real0/fft_direct/butterfly0_0
      settings: AttributesFormatString=twiddle_general
      mask TypeOptions quantization: Truncate | Round  (unbiased: +/- Inf) | Round  (unbiased: Even Values)
      mask TypeOptions overflow: Wrap | Saturate | Error
      mask Callback use_hdl:
      | % make mutually exclusive with use_embedded.
      | 
      | use_hdl = get_param(gcb, 'use_hdl');
      | if strcmp(use_hdl, 'on'),
      |     set_param_state(gcb, 'use_embedded', 'off');
      | else
      |     set_param_state(gcb, 'use_embedded', 'on');
      | end
      mask Callback use_embedded:
      | % make mutually exclusive with use_hdl.
      | 
      | use_hdl = get_param(gcb, 'use_embedded');
      | if strcmp(use_hdl, 'on'),
      |     set_param_state(gcb, 'use_hdl', 'off');
      | else
      |     set_param_state(gcb, 'use_hdl', 'on');
      | end
      mask Callback hardcode_shifts:
      | hardcode_shifts = get_param(gcb, 'hardcode_shifts');
      | set_param_state(gcb, 'downshift', hardcode_shifts);
      mask Callback dsp48_adders: %fft_callback_dsp48_adders(gcb);
  combine : xbsIndex_r4/Logical  logical_function=OR  inputs=8  en=off  latency=2  precision=Full  arith_type=Unsigned  n_bits=16  bin_pt=0  align_bp=on  dbl_ovrd=off  block_type=logical
  of_0 : xbsIndex_r4/Delay  rst=off  infoeditControl=Selection of Reset will increase slice count due to use of real FFs and instead of SRLs  en=off  latency=0  dbl_ovrd=off  reg_retiming=on  block_type=delay
  of_1 : xbsIndex_r4/Concat  num_inputs=2  dbl_ovrd=off  block_type=concat
  of_2 : xbsIndex_r4/Concat  num_inputs=4  dbl_ovrd=off  block_type=concat
  of_3 : xbsIndex_r4/Concat  num_inputs=8  dbl_ovrd=off  block_type=concat
  of_expand : Subsystem  AncestorBlock=casper_library_flow_control/bus_expand  mask(bus_expand){mode=divisions of equal size, outputNum=8, outputWidth=1, outputBinaryPt=0, outputArithmeticType=0, show_format=off, outputToWorkspace=off, variablePrefix=out, outputToModelAsWell=on}
      mask prompts: outputNum "Number of outputs:"; outputWidth "Output width:"; outputBinaryPt "Output binary point position:"; outputArithmeticType "Output arithmetic type (ufix=0, fix=1, bool=2):"; show_format "Print format string?"; outputToWorkspace "Output to workspace?"; variablePrefix "Variable name prefix:"; outputToModelAsWell "Output to model as well?"
      mask help:
      | Expands a 'bus' made using a bus_create or Xilinx
      | concat block.
      | The input will be divided into the specified number
      | of outputs with the specified arithmetic types.
      | Divisions are made from most significant bit.
      mask init: same as spec2in_8192ch_1080mhz/dout0_0/munge_in/split
      settings: AttributesFormatString=8 outputs
      mask TypeOptions mode: divisions of equal size | divisions of arbitrary size
      mask Callback mode: bus_expand_callback();
      mask Callback outputWidth: bus_expand_callback();
      mask Callback outputToWorkspace: bus_expand_callback();
  of_or : xbsIndex_r4/Logical  logical_function=OR  inputs=4  en=off  latency=2  precision=Full  arith_type=Unsigned  n_bits=16  bin_pt=0  align_bp=on  dbl_ovrd=off  block_type=logical
  slice0 : xbsIndex_r4/Slice  nbits=1  boolean_output=on  mode=Lower Bit Location + Width  bit1=0  base1=MSB of Input  bit0=0  base0=LSB of Input  dbl_ovrd=off  block_type=slice
  slice1 : xbsIndex_r4/Slice  nbits=1  boolean_output=on  mode=Lower Bit Location + Width  bit1=0  base1=MSB of Input  bit0=1  base0=LSB of Input  dbl_ovrd=off  block_type=slice
  slice2 : xbsIndex_r4/Slice  nbits=1  boolean_output=on  mode=Lower Bit Location + Width  bit1=0  base1=MSB of Input  bit0=2  base0=LSB of Input  dbl_ovrd=off  block_type=slice
  slice3 : xbsIndex_r4/Slice  nbits=1  boolean_output=on  mode=Lower Bit Location + Width  bit1=0  base1=MSB of Input  bit0=3  base0=LSB of Input  dbl_ovrd=off  block_type=slice
  synct{1..7} (7 alike) : Terminator
  sync_out : Outport
  out00 : Outport  Port=2
  out01 : Outport  Port=3
  out02 : Outport  Port=4
  out03 : Outport  Port=5
  out04 : Outport  Port=6
  out05 : Outport  Port=7
  out06 : Outport  Port=8
  out07 : Outport  Port=9
  out08 : Outport  Port=10
  out09 : Outport  Port=11
  out010 : Outport  Port=12
  out011 : Outport  Port=13
  out012 : Outport  Port=14
  out013 : Outport  Port=15
  out014 : Outport  Port=16
  out015 : Outport  Port=17
  of : Outport  Port=18
  wires:
  in00/1 -> abus/1(in1)
  adebus3_0/1(msb_lsb_out1) -> out00/1
  in01/1 -> abus/2(in2)
  adebus3_4/1(msb_lsb_out1) -> out01/1
  in02/1 -> abus/3(in3)
  adebus3_2/1(msb_lsb_out1) -> out02/1
  in03/1 -> abus/4(in4)
  adebus3_6/1(msb_lsb_out1) -> out03/1
  in04/1 -> abus/5(in5)
  adebus3_1/1(msb_lsb_out1) -> out04/1
  in05/1 -> abus/6(in6)
  adebus3_5/1(msb_lsb_out1) -> out05/1
  in06/1 -> abus/7(in7)
  adebus3_3/1(msb_lsb_out1) -> out06/1
  in07/1 -> abus/8(in8)
  adebus3_7/1(msb_lsb_out1) -> out07/1
  in08/1 -> bbus/1(in1)
  bdebus3_0/1(msb_lsb_out1) -> out08/1
  in09/1 -> bbus/2(in2)
  bdebus3_4/1(msb_lsb_out1) -> out09/1
  in010/1 -> bbus/3(in3)
  bdebus3_2/1(msb_lsb_out1) -> out010/1
  in011/1 -> bbus/4(in4)
  bdebus3_6/1(msb_lsb_out1) -> out011/1
  in012/1 -> bbus/5(in5)
  bdebus3_1/1(msb_lsb_out1) -> out012/1
  in013/1 -> bbus/6(in6)
  bdebus3_5/1(msb_lsb_out1) -> out013/1
  in014/1 -> bbus/7(in7)
  bdebus3_3/1(msb_lsb_out1) -> out014/1
  in015/1 -> bbus/8(in8)
  bdebus3_7/1(msb_lsb_out1) -> out015/1
  of_or/1 -> of_expand/1(bus_in)
  of_expand/1(msb_out8) -> combine/1
  of_expand/2(out7) -> combine/2
  of_expand/3(out6) -> combine/3
  of_expand/4(out5) -> combine/4
  of_expand/5(out4) -> combine/5
  of_expand/6(out3) -> combine/6
  of_expand/7(out2) -> combine/7
  of_expand/8(lsb_out1) -> combine/8
  combine/1 -> of/1
  shift/1 -> slice0/1, slice1/1, slice2/1, slice3/1
  of_0/1 -> of_or/1
  sync/1 -> butterfly0_0/3(sync_in)
  slice0/1 -> butterfly0_0/4(shift)
  abus/1(bus_out) -> butterfly0_0/1(a)
  bbus/1(bus_out) -> butterfly0_0/2(b)
  butterfly0_0/3(of) -> of_0/1
  butterfly0_0/1(a+bw) -> adebus0_0/1(bus_in)
  butterfly0_0/2(a-bw) -> bdebus0_0/1(bus_in)
  of_1/1 -> of_or/2
  butterfly0_0/4(sync_out) -> butterfly1_0/3(sync_in), butterfly1_1/3(sync_in)
  slice1/1 -> butterfly1_0/4(shift), butterfly1_1/4(shift)
  adebus0_0/1(msb_out2) -> butterfly1_0/1(a)
  adebus0_0/2(lsb_out1) -> butterfly1_0/2(b)
  butterfly1_0/3(of) -> of_1/1
  butterfly1_0/1(a+bw) -> adebus1_0/1(bus_in)
  butterfly1_0/2(a-bw) -> bdebus1_0/1(bus_in)
  bdebus0_0/1(msb_out2) -> butterfly1_1/1(a)
  bdebus0_0/2(lsb_out1) -> butterfly1_1/2(b)
  butterfly1_1/3(of) -> of_1/2
  butterfly1_1/1(a+bw) -> adebus1_1/1(bus_in)
  butterfly1_1/2(a-bw) -> bdebus1_1/1(bus_in)
  of_2/1 -> of_or/3
  butterfly1_0/4(sync_out) -> butterfly2_0/3(sync_in), butterfly2_1/3(sync_in)
  slice2/1 -> butterfly2_0/4(shift), butterfly2_1/4(shift), butterfly2_2/4(shift), butterfly2_3/4(shift)
  adebus1_0/1(msb_out2) -> butterfly2_0/1(a)
  adebus1_0/2(lsb_out1) -> butterfly2_0/2(b)
  butterfly2_0/3(of) -> of_2/1
  butterfly2_0/1(a+bw) -> adebus2_0/1(bus_in)
  butterfly2_0/2(a-bw) -> bdebus2_0/1(bus_in)
  bdebus1_0/1(msb_out2) -> butterfly2_1/1(a)
  bdebus1_0/2(lsb_out1) -> butterfly2_1/2(b)
  butterfly2_1/3(of) -> of_2/2
  butterfly2_1/1(a+bw) -> adebus2_1/1(bus_in)
  butterfly2_1/2(a-bw) -> bdebus2_1/1(bus_in)
  butterfly1_1/4(sync_out) -> butterfly2_2/3(sync_in), butterfly2_3/3(sync_in)
  adebus1_1/1(msb_out2) -> butterfly2_2/1(a)
  adebus1_1/2(lsb_out1) -> butterfly2_2/2(b)
  butterfly2_2/3(of) -> of_2/3
  butterfly2_2/1(a+bw) -> adebus2_2/1(bus_in)
  butterfly2_2/2(a-bw) -> bdebus2_2/1(bus_in)
  bdebus1_1/1(msb_out2) -> butterfly2_3/1(a)
  bdebus1_1/2(lsb_out1) -> butterfly2_3/2(b)
  butterfly2_3/3(of) -> of_2/4
  butterfly2_3/1(a+bw) -> adebus2_3/1(bus_in)
  butterfly2_3/2(a-bw) -> bdebus2_3/1(bus_in)
  of_3/1 -> of_or/4
  butterfly2_0/4(sync_out) -> butterfly3_0/3(sync_in), butterfly3_1/3(sync_in)
  butterfly3_0/4(sync_out) -> sync_out/1
  slice3/1 -> butterfly3_0/4(shift), butterfly3_1/4(shift), butterfly3_2/4(shift), butterfly3_3/4(shift), butterfly3_4/4(shift), butterfly3_5/4(shift), butterfly3_6/4(shift), butterfly3_7/4(shift)
  adebus2_0/1(msb_out2) -> butterfly3_0/1(a)
  adebus2_0/2(lsb_out1) -> butterfly3_0/2(b)
  butterfly3_0/3(of) -> of_3/1
  butterfly3_1/4(sync_out) -> synct1/1
  bdebus2_0/1(msb_out2) -> butterfly3_1/1(a)
  bdebus2_0/2(lsb_out1) -> butterfly3_1/2(b)
  butterfly3_1/3(of) -> of_3/2
  butterfly2_1/4(sync_out) -> butterfly3_2/3(sync_in), butterfly3_3/3(sync_in)
  butterfly3_2/4(sync_out) -> synct2/1
  adebus2_1/1(msb_out2) -> butterfly3_2/1(a)
  adebus2_1/2(lsb_out1) -> butterfly3_2/2(b)
  butterfly3_2/3(of) -> of_3/3
  butterfly3_3/4(sync_out) -> synct3/1
  bdebus2_1/1(msb_out2) -> butterfly3_3/1(a)
  bdebus2_1/2(lsb_out1) -> butterfly3_3/2(b)
  butterfly3_3/3(of) -> of_3/4
  butterfly2_2/4(sync_out) -> butterfly3_4/3(sync_in), butterfly3_5/3(sync_in)
  butterfly3_4/4(sync_out) -> synct4/1
  adebus2_2/1(msb_out2) -> butterfly3_4/1(a)
  adebus2_2/2(lsb_out1) -> butterfly3_4/2(b)
  butterfly3_4/3(of) -> of_3/5
  butterfly3_5/4(sync_out) -> synct5/1
  bdebus2_2/1(msb_out2) -> butterfly3_5/1(a)
  bdebus2_2/2(lsb_out1) -> butterfly3_5/2(b)
  butterfly3_5/3(of) -> of_3/6
  butterfly2_3/4(sync_out) -> butterfly3_6/3(sync_in), butterfly3_7/3(sync_in)
  butterfly3_6/4(sync_out) -> synct6/1
  adebus2_3/1(msb_out2) -> butterfly3_6/1(a)
  adebus2_3/2(lsb_out1) -> butterfly3_6/2(b)
  butterfly3_6/3(of) -> of_3/7
  butterfly3_7/4(sync_out) -> synct7/1
  bdebus2_3/1(msb_out2) -> butterfly3_7/1(a)
  bdebus2_3/2(lsb_out1) -> butterfly3_7/2(b)
  butterfly3_7/3(of) -> of_3/8
  butterfly3_0/1(a+bw) -> adebus3_0/1(bus_in)
  butterfly3_0/2(a-bw) -> bdebus3_0/1(bus_in)
  butterfly3_1/1(a+bw) -> adebus3_1/1(bus_in)
  butterfly3_1/2(a-bw) -> bdebus3_1/1(bus_in)
  butterfly3_2/1(a+bw) -> adebus3_2/1(bus_in)
  butterfly3_2/2(a-bw) -> bdebus3_2/1(bus_in)
  butterfly3_3/1(a+bw) -> adebus3_3/1(bus_in)
  butterfly3_3/2(a-bw) -> bdebus3_3/1(bus_in)
  butterfly3_4/1(a+bw) -> adebus3_4/1(bus_in)
  butterfly3_4/2(a-bw) -> bdebus3_4/1(bus_in)
  butterfly3_5/1(a+bw) -> adebus3_5/1(bus_in)
  butterfly3_5/2(a-bw) -> bdebus3_5/1(bus_in)
  butterfly3_6/1(a+bw) -> adebus3_6/1(bus_in)
  butterfly3_6/2(a-bw) -> bdebus3_6/1(bus_in)
  butterfly3_7/1(a+bw) -> adebus3_7/1(bus_in)
  butterfly3_7/2(a-bw) -> bdebus3_7/1(bus_in)
